simulink model "ofdm_txrx_supermimo_coded"  (Simulink 7.7)
settings: Solver=VariableStepDiscrete  SolverMode=SingleTasking  StartTime=0.0  StopTime=2*simOnly_simLength  RelTol=1e-3
PostLoadFcn:
  | ofdm_rx_supermimo_init
InitFcn:
  | ofdm_rx_supermimo_init
StartFcn:
  | ofdm_rx_supermimo_init
values from neighbouring files (symbols this model uses):
  ActionID_AFTransmit = 31    [ofdm_tx_supermimo_init.m]
  ActionID_Disabled = 0    [ofdm_tx_supermimo_init.m]
  ActionID_SetFlagA = 62    [ofdm_tx_supermimo_init.m]
  ActionID_SetFlagB = 61    [ofdm_tx_supermimo_init.m]
  BER_RAM_init_size = 2048    [ofdm_tx_supermimo_init.m]
  channel_h = [1 0; 1 0] * 0.3    [ofdm_tx_supermimo_init.m]
  CPLength = 16    [ofdm_tx_supermimo_init.m]
  longCorr_coef_i = [-4*real(LTS) 0]    [ofdm_rx_supermimo_init.m]
  longCorr_coef_q = [4*imag(LTS) 0]    [ofdm_rx_supermimo_init.m]
  max_CP_length = 16    [ofdm_tx_supermimo_init.m]
  max_num_baseRateSymbols = 31    [ofdm_tx_supermimo_init.m]
  max_num_subcarriers = 64    [ofdm_tx_supermimo_init.m]
  max_num_trainingSymbols = 15    [ofdm_tx_supermimo_init.m]
  max_numBytes = 16384    [ofdm_tx_supermimo_init.m]
  max_OFDM_symbols = 1023    [ofdm_tx_supermimo_init.m]
  modConstellation_qam16 = .75*[1 3 -1 -3]./3    [ofdm_tx_supermimo_init.m]
  modConstellation_qam64 = 0.875*[3 1 5 7 -3 -1 -5 -7]./7    [ofdm_tx_supermimo_init.m]
  modConstellation_qpsk = [1 -1]./sqrt(2)    [ofdm_tx_supermimo_init.m]
  numBaseRateSymbols = numBaseRateSymbols *2    [ofdm_tx_supermimo_init.m]
  numSubcarriers = 64    [ofdm_tx_supermimo_init.m]
  numTrainingSymbols = 2    [ofdm_tx_supermimo_init.m]
  pktTiming_count_payloadStart = pktTiming_count_lsStart + pktTiming_ls_num*pktTiming_ls_length    [ofdm_rx_supermimo_init.m]
  preamble = preamble_scale*[shortsyms_10 longsyms_2]    [ofdm_tx_supermimo_init.m]
  preamble_ROM = preamble_I_ROM + preamble_Q_ROM*2^16    [ofdm_tx_supermimo_init.m]
  RAM_init_size = max_numBytes    (= 16384)    [ofdm_tx_supermimo_init.m]
  RxDataScrambling_Seq = [40 198 78 63 82 173 102 245 48 111 172 115 147 230 216 93 72 65 62 2 205 242 122 90 128 83 105 97 73 10 5 252]    [ofdm_tx_supermimo_init.m]
  shortSymbol_time = shortSymbol_time(1:16).'    [ofdm_tx_supermimo_init.m]
  simOnly_numSamples = length(preamble)+( (numSubcarriers+CPLength)*(numTrainingSymbols + numBaseRateSymbols + numFullRateSymbols/2) )    [ofdm_tx_supermimo_init.m]
  train = [train train]    [ofdm_tx_supermimo_init.m]
  TxDataScrambling_Seq = [40 198 78 63 82 173 102 245 48 111 172 115 147 230 216 93 72 65 62 2 205 242 122 90 128 83 105 97 73 10 5 252]    [ofdm_tx_supermimo_init.m]
undefined symbols (not defined in the file or in its folder): AntA, AntB, BaseRate, Down, gcbh, numBuffs, numBytes, Up, xlBlockLoadCallback, xlGetNormalizedPeriod, xlipgetpartsetting
libraries: xbsIndex_r4 x5430
(part 11 of 49)

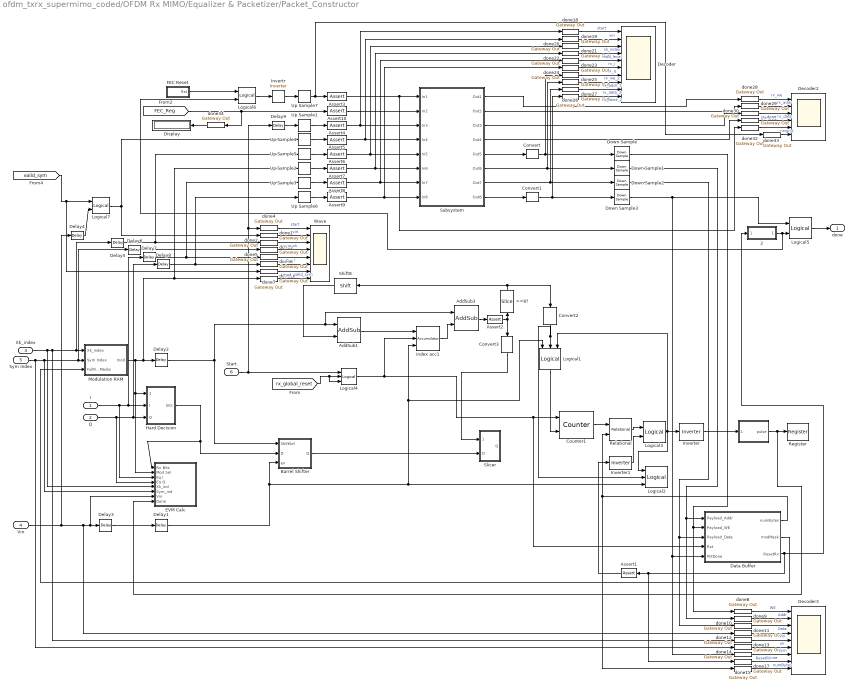
== system ofdm_txrx_supermimo_coded/OFDM Rx MIMO/Equalizer & Packetizer/Packet_Constructor ==
  I : Inport
  Q : Inport  Port=2
  Xk_index : Inport  Port=3
  Vin : Inport  Port=4
  Sym Index : Inport  Port=5
  Start : Inport  Port=6
  "  " : Subsystem  mask{period=1}
  "  2" : Subsystem  mask{period=1}
  >=8? : xbsIndex_r4/Slice  nbits=1  boolean_output=off  mode=Lower Bit Location + Width  bit1=0  base1=MSB of Input  bit0=3  base0=LSB of Input  dbl_ovrd=off  block_type=slice
      settings: infoedit="Extracts a given range of bits from each input sample and presents it at the output.  The output type is ordinarily unsigned with binary point at zero, but can be Boolean when the slice is one bit wide.<P><P>Hardware notes: In hardware this block costs nothing."  block_version=VER_STRING_GOES_HERE
  AddSub1 : xbsIndex_r4/AddSub  mode=Subtraction  use_carryin=off  use_carryout=off  en=off  latency=0  precision=User Defined  arith_type=Unsigned  n_bits=4  bin_pt=0  quantization=Truncate  overflow=Wrap  dbl_ovrd=off  use_behavioral_HDL=on  hw_selection=Fabric  pipelined=off  use_rpm=on  block_type=addsub
      settings: xl_area=[2 0 0 4 0 0 0]  block_version=VER_STRING_GOES_HERE
  AddSub3 : xbsIndex_r4/AddSub  mode=Addition  use_carryin=off  use_carryout=off  en=off  latency=0  precision=User Defined  arith_type=Unsigned  n_bits=4  bin_pt=0  quantization=Truncate  overflow=Wrap  dbl_ovrd=off  use_behavioral_HDL=on  hw_selection=Fabric  pipelined=off  use_rpm=on  block_type=addsub
      settings: xl_area=[2 0 0 4 0 0 0]  block_version=VER_STRING_GOES_HERE
  Assert1 : xbsIndex_r4/Assert  assert_type=off  type_source=Explicitly  gui_display_data_type=Fixed-point  arith_type="Signed  (2's comp)"  n_bits=16  bin_pt=14  preci_type=Single  exp_width=8  frac_width=24  assert_rate=on  rate_source=Explicitly  period=2  output_port=on  block_type=assert
      settings: infoedit=Asserts a user-defined sample rate and/or type on a signal.<P><P>Hardware notes: In hardware this block costs nothing.  block_version=VER_STRING_GOES_HERE
  Assert{3..10} (8 alike) : xbsIndex_r4/Assert  assert_type=off  type_source=Explicitly  gui_display_data_type=Fixed-point  arith_type="Signed  (2's comp)"  n_bits=16  bin_pt=14  preci_type=Single  exp_width=8  frac_width=24  assert_rate=on  rate_source=Explicitly  period=1  output_port=on  block_type=assert
      settings: infoedit=Asserts a user-defined sample rate and/or type on a signal.<P><P>Hardware notes: In hardware this block costs nothing.  block_version=10.1.3
  Assert2 : xbsIndex_r4/Assert  assert_type=off  type_source=Explicitly  gui_display_data_type=Fixed-point  arith_type="Signed  (2's comp)"  n_bits=16  bin_pt=14  preci_type=Single  exp_width=8  frac_width=24  assert_rate=on  rate_source=Explicitly  period=2  output_port=on  block_type=assert
      settings: infoedit=Asserts a user-defined sample rate and/or type on a signal.<P><P>Hardware notes: In hardware this block costs nothing.  block_version=VER_STRING_GOES_HERE
  Barrel Shifter : Subsystem
  Convert : xbsIndex_r4/Convert  gui_display_data_type=Boolean  arith_type=Boolean  n_bits=16  bin_pt=14  float_type=Single  exp_bits=8  fraction_bits=24  quantization=Truncate  overflow=Wrap  en=off  latency=0  dbl_ovrd=off  pipeline=off  block_type=convert
  Convert1 : xbsIndex_r4/Convert  gui_display_data_type=Boolean  arith_type=Boolean  n_bits=16  bin_pt=14  float_type=Single  exp_bits=8  fraction_bits=24  quantization=Truncate  overflow=Wrap  en=off  latency=0  dbl_ovrd=off  pipeline=off  block_type=convert
  Convert2 : xbsIndex_r4/Convert  gui_display_data_type=Fixed-point  arith_type=Boolean  n_bits=8  bin_pt=6  float_type=Single  exp_bits=8  fraction_bits=24  quantization=Truncate  overflow=Wrap  en=off  latency=0  dbl_ovrd=off  pipeline=off  block_type=convert
      settings: xl_area=[0 0 0 0 0 0 0]  block_version=VER_STRING_GOES_HERE
  Convert3 : xbsIndex_r4/Convert  gui_display_data_type=Fixed-point  arith_type=Unsigned  n_bits=3  bin_pt=0  float_type=Single  exp_bits=8  fraction_bits=24  quantization=Truncate  overflow=Wrap  en=off  latency=0  dbl_ovrd=off  pipeline=off  block_type=convert
      settings: xl_area=[0 0 0 0 0 0 0]  block_version=VER_STRING_GOES_HERE
  Counter1 : xbsIndex_r4/Counter  cnt_type=Free Running  cnt_to=Inf  operation=Up  start_count=0  cnt_by_val=1  arith_type=Unsigned  n_bits=ceil(log2(RAM_init_size))  bin_pt=0  load_pin=off  rst=on  en=on  explicit_period=off  period=2  dbl_ovrd=off  use_behavioral_HDL=off  implementation=Fabric  use_rpm=on  block_type=counter
      settings: xl_area=[5 10 0 10 0 0 0]  block_version=VER_STRING_GOES_HERE
  Data Buffer : Subsystem
  Decoder : Scope  NumInputPorts=10
      settings: TimeRange=14000  YMin=-1~-1~0~-1~-0.75~-1~0~0~0~-1  YMax=2~2~75~5~0.75~1~2~150~300~2  SaveName=ScopeData33
  Decoder2 : Scope  NumInputPorts=6
      settings: TimeRange=15000  YMin=-1~-1~-1~-1~-1~-1  YMax=2~2~1~2~2~2  SaveName=ScopeData35
  Decoder3 : Scope  NumInputPorts=9
      settings: TimeRange=6724.590163934425  YMin=-1~-1~-1~-1~0~0~0~-5~-5  YMax=2~2~1~2~1~3500~1~5~5  SaveName=ScopeData24
  Delay{1..9} (9 alike) : xbsIndex_r4/Delay  rst=off  infoeditControl=Selection of Reset will increase slice count due to use of real FFs and instead of SRLs  en=off  latency=1  dbl_ovrd=off  reg_retiming=off  block_type=delay
      settings: infoedit=Hardware notes: A delay line is a chain, each link of which is an SRL16 followed by a flip-flop. If register retiming is enabled, the delay line is a chain of flip-flops.  xl_area=[1 1 0 0 0 0 0]  block_version=VER_STRING_GOES_HERE
  Display : Display
  Down Sample{,1..3} (4 alike) : xbsIndex_r4/Down Sample  sample_ratio=2  sample_phase="Last Value of Frame  (most efficient)"  en=off  latency=1  dbl_ovrd=off  block_type=dsamp
      settings: block_version=10.1.3
  EVM Calc : Subsystem
  FEC Reset : Subsystem
  Hard Decision : Subsystem
  Inverter : xbsIndex_r4/Inverter  en=off  latency=0  dbl_ovrd=off  block_type=inv
      settings: xl_area=[0 0 0 0 0 0 0]  block_version=VER_STRING_GOES_HERE
  Inverter1 : xbsIndex_r4/Inverter  en=off  latency=0  dbl_ovrd=off  block_type=inv
      settings: xl_area=[0 0 0 0 0 0 0]  block_version=VER_STRING_GOES_HERE
  Invertr : xbsIndex_r4/Inverter  en=off  latency=0  dbl_ovrd=off  block_type=inv
  Logical1 : xbsIndex_r4/Logical  logical_function=AND  inputs=3  en=off  latency=0  precision=Full  arith_type=Unsigned  n_bits=8  bin_pt=2  align_bp=on  dbl_ovrd=off  block_type=logical
      settings: xl_area=[1 0 0 1 0 0 0]  block_version=VER_STRING_GOES_HERE
  Logical2 : xbsIndex_r4/Logical  logical_function=AND  inputs=3  en=off  latency=0  precision=Full  arith_type=Unsigned  n_bits=8  bin_pt=2  align_bp=on  dbl_ovrd=off  block_type=logical
      settings: xl_area=[1 0 0 1 0 0 0]  block_version=VER_STRING_GOES_HERE
  Logical3 : xbsIndex_r4/Logical  logical_function=AND  inputs=2  en=off  latency=0  precision=Full  arith_type=Unsigned  n_bits=8  bin_pt=2  align_bp=on  dbl_ovrd=off  block_type=logical
      settings: xl_area=[1 0 0 1 0 0 0]  block_version=VER_STRING_GOES_HERE
  Logical4 : xbsIndex_r4/Logical  logical_function=OR  inputs=3  en=off  latency=0  precision=Full  arith_type=Unsigned  n_bits=8  bin_pt=2  align_bp=on  dbl_ovrd=off  block_type=logical
      settings: xl_area=[4 0 0 4 0 0 0]  block_version=VER_STRING_GOES_HERE
  Logical5 : xbsIndex_r4/Logical  logical_function=OR  inputs=2  en=off  latency=0  precision=Full  arith_type=Unsigned  n_bits=8  bin_pt=2  align_bp=on  dbl_ovrd=off  block_type=logical
      settings: xl_area=[1 0 0 1 0 0 0]  block_version=VER_STRING_GOES_HERE
  Logical6 : xbsIndex_r4/Logical  logical_function=OR  inputs=2  en=off  latency=0  precision=Full  arith_type=Unsigned  n_bits=8  bin_pt=2  align_bp=on  dbl_ovrd=off  block_type=logical
      settings: xl_area=[1 0 0 1 0 0 0]  block_version=VER_STRING_GOES_HERE
  Logical7 : xbsIndex_r4/Logical  logical_function=AND  inputs=2  en=off  latency=0  precision=Full  arith_type=Unsigned  n_bits=16  bin_pt=0  align_bp=on  dbl_ovrd=off  block_type=logical
      settings: block_version=VER_STRING_GOES_HERE
  Modulation RAM : Subsystem
  Register : xbsIndex_r4/Register  init=0  rst=off  en=off  dbl_ovrd=off  block_type=register
      settings: block_version=8.2.02
  Relational : xbsIndex_r4/Relational  mode=a<b  en=off  latency=0  dbl_ovrd=off  block_type=relational
      settings: xl_area=[6 0 0 11 0 0 0]  block_version=VER_STRING_GOES_HERE
  Shift8 : xbsIndex_r4/Shift  shift_dir=Left  shift_bits=3  en=off  latency=0  precision=User Defined  arith_type=Unsigned  n_bits=4  bin_pt=0  quantization=Truncate  overflow=Wrap  dbl_ovrd=off  block_type=shift
      settings: xl_area=[0 0 0 0 0 0 0]  block_version=VER_STRING_GOES_HERE
  Slicer : Subsystem
  Subsystem : Subsystem
  Up Sample1 : xbsIndex_r4/Up Sample  sample_ratio=4  copy_samples=off  en=off  latency=0  dbl_ovrd=off  block_type=usamp
      settings: infoedit="Up samples input data.  Inserted values  can be zeros or copies of the most recent input sample.<P><P>Hardware notes: No hardware is needed if inserted values are copies of the input sample; otherwise, a mux and single bit flip-flop are used."  block_version=10.1.3
  Up Sample{2..3,6..7} (4 alike) : xbsIndex_r4/Up Sample  sample_ratio=2  copy_samples=on  en=off  latency=0  dbl_ovrd=off  block_type=usamp
      settings: infoedit="Up samples input data.  Inserted values  can be zeros or copies of the most recent input sample.<P><P>Hardware notes: No hardware is needed if inserted values are copies of the input sample; otherwise, a mux and single bit flip-flop are used."  block_version=10.1.3
  Up Sample4 : xbsIndex_r4/Up Sample  sample_ratio=2  copy_samples=off  en=off  latency=0  dbl_ovrd=off  block_type=usamp
      settings: infoedit="Up samples input data.  Inserted values  can be zeros or copies of the most recent input sample.<P><P>Hardware notes: No hardware is needed if inserted values are copies of the input sample; otherwise, a mux and single bit flip-flop are used."  block_version=10.1.3
  Up Sample5 : xbsIndex_r4/Up Sample  sample_ratio=2  copy_samples=off  en=off  latency=0  dbl_ovrd=off  block_type=usamp
      settings: infoedit="Up samples input data.  Inserted values  can be zeros or copies of the most recent input sample.<P><P>Hardware notes: No hardware is needed if inserted values are copies of the input sample; otherwise, a mux and single bit flip-flop are used."  block_version=10.1.3
  Wave : Scope  NumInputPorts=8
      settings: TimeRange=18000  YMin=-1~-1~-1~24.7~0.00168152~-0.75~0~0  YMax=2~2~1~27.3~0.00185852~0.75~2~5  SaveName=ScopeData21
  done{1..34} (34 alike) : xbsIndex_r4/Gateway Out  inherit_from_input=off  hdl_port=off  timing_constraint=None  locs_specified=off  LOCs={}  UseAsDAC=off  DACChannel='1'  block_type=gatewayout
      settings: infoedit="Gateway out block.  Converts Xilinx fixed point inputs into ouputs of type Simulink integer, double, or fixed point.<P><P>Hardware notes:  In hardware these blocks become top level output ports or are discarded, depending on how they are configured."  xl_area=[0 0 0 0 1 0 0]  block_version=VER_STRING_GOES_HERE
  index acc1 : xbsIndex_r4/Accumulator  operation=Add  n_bits=4  overflow=Wrap  scale=1  rst=on  hasbypass=off  en=on  dbl_ovrd=off  use_behavioral_HDL=on  implementation=Fabric  block_type=accum
      settings: infoedit="Adder or subtractor-based accumulator.   Output type and binary point position match the input.<P><P>Hardware notes: When \"Reinitialize with input 'b' on reset\" is selected, the accumulator is forced to run at the system rate even if the input 'b' is running at a slower rate."  xl_area=[2 4 0 4 0 0 0]  block_version=VER_STRING_GOES_HERE
  done : Outport
  wires:
  Delay2/1 -> AddSub1/1, AddSub3/1, Barrel Shifter/1(ShiftSel)
  Data Buffer/1(numBytes) -> Relational/2, done17/1
  Logical4/1 -> index acc1/2, Counter1/1, Data Buffer/4(Rst)
  AddSub1/1 -> index acc1/1
  Shift8/1 -> AddSub1/2
  index acc1/1 -> AddSub3/2
  AddSub3/1 -> Assert2/1
  >=8?/1 -> Shift8/1, Convert2/1
  Barrel Shifter/1(Q) -> Slicer/2(D)
  "  "/1(pulse) -> Register/1, EVM Calc/8(Done)
  Inverter/1 -> "  "/1(signal)
  Convert2/1 -> Logical1/2, Logical2/2
  Logical1/1 -> Counter1/2
  Logical3/1 -> Logical2/1, Logical1/3, Inverter/1
  Counter1/1 -> Relational/1
  Convert3/1 -> Slicer/1(SliceSel)
  Modulation RAM/1(mod) -> Hard Decision/1(Mod Sel), EVM Calc/2(Mod Sel), Delay2/1, done16/1, Up Sample2/1
  Xk_index/1 -> EVM Calc/5(Xk_ind), Modulation RAM/1(Xk_index), Delay5/1, done12/1
  Sym Index/1 -> EVM Calc/6(Sym_ind), Delay6/1, Modulation RAM/2(Sym Index), done13/1
  Hard Decision/1(bits) -> Barrel Shifter/2(D), EVM Calc/1(Rx Bits)
  I/1 -> EVM Calc/3(Eq I), Hard Decision/2(I), Delay7/1
  Q/1 -> EVM Calc/4(Eq Q), Hard Decision/3(Q), Delay8/1
  Start/1 -> Logical4/1, done4/1, Delay9/1
  [rx_global_reset] -> Logical4/2, Logical4/3
  Assert2/1 -> Convert3/1, >=8?/1
  Vin/1 -> Delay3/1, EVM Calc/7(Vin), done11/1, Delay4/1
  Delay1/1 -> Barrel Shifter/3(en), index acc1/3, Logical1/1, Logical2/3
  Delay3/1 -> Delay1/1
  Data Buffer/2(modMask) -> Modulation RAM/3(FullRateMasks)
  Relational/1 -> Logical3/1
  Inverter1/1 -> Logical3/2
  Assert1/1 -> Inverter1/1
  Data Buffer/3(ResetRx) -> Assert1/1, done15/1, "  2"/1(signal)
  done4/1 -> Wave/1  "start"
  done1/1 -> Wave/2  "vin"
  done2/1 -> Wave/3  "xk"
  done3/1 -> Wave/4  "sym"
  done5/1 -> Wave/5  "I"
  done6/1 -> Wave/6  "Q"
  done7/1 -> Wave/7  "valid_sym"
  done8/1 -> Decoder3/1  "WE"
  done9/1 -> Decoder3/2  "Addr"
  done10/1 -> Decoder3/3  "Data"
  done11/1 -> Decoder3/4  "Vin"
  done12/1 -> Decoder3/5  "xk"
  done13/1 -> Decoder3/6  "Sym"
  done14/1 -> Decoder3/7  "done"
  done15/1 -> Decoder3/8  "ResetRx"
  done16/1 -> Wave/8  "mod"
  done17/1 -> Decoder3/9  "numBytes"
  Delay5/1 -> done2/1, Up Sample5/1
  Delay6/1 -> done3/1
  Delay7/1 -> done5/1, Up Sample3/1
  Delay8/1 -> done6/1, Up Sample6/1
  Invertr/1 -> Up Sample7/1
  Up Sample1/1 -> Assert4/1
  Assert4/1 -> done18/1, Subsystem/3(In3)
  Delay9/1 -> Up Sample1/1
  Up Sample4/1 -> Assert5/1
  Assert5/1 -> done19/1, Subsystem/4(In4)
  Assert6/1 -> done20/1, Subsystem/5(In5)
  Up Sample5/1 -> Assert6/1
  Up Sample2/1 -> Assert7/1
  Assert7/1 -> done21/1, Subsystem/6(In6)
  Up Sample3/1 -> Assert8/1
  Assert8/1 -> done22/1, Subsystem/7(In7)
  Up Sample6/1 -> Assert9/1
  Assert9/1 -> done23/1, Subsystem/8(In8)
  [FEC_Reg] -> Assert10/1, done34/1
  Assert10/1 -> Subsystem/2(In2)
  Subsystem/5(Out5) -> Convert/1
  Subsystem/6(Out6) -> done25/1, Down Sample1/1
  Subsystem/7(Out7) -> done26/1, Down Sample2/1
  done18/1 -> Decoder/1  "start"
  done20/1 -> Decoder/3  "xk_index"
  done21/1 -> Decoder/4  "mod_level"
  done22/1 -> Decoder/5  "rx_i"
  done23/1 -> Decoder/6  "rx_q"
  done24/1 -> Decoder/7  "rx_we_2"
  done25/1 -> Decoder/8  "rx_addr_2"
  done26/1 -> Decoder/9  "rx_data_2"
  done27/1 -> Decoder/10  "rx_done_2"
  done19/1 -> Decoder/2  "vin"
  done28/1 -> Decoder2/1  "rx_we"
  Subsystem/1(Out1) -> done28/1
  Subsystem/2(Out2) -> done29/1
  done29/1 -> Decoder2/2  "rx_addr"
  done30/1 -> Decoder2/3  "rx_data"
  Subsystem/3(Out3) -> done30/1
  done31/1 -> Decoder2/4  "rx_done"
  Subsystem/4(Out4) -> done31/1
  done32/1 -> Decoder2/5  "nrst"
  Down Sample/1 -> Data Buffer/2(Payload_WE), done8/1
  Down Sample1/1 -> Data Buffer/1(Payload_Addr), done9/1
  Down Sample2/1 -> Data Buffer/3(Payload_Data), done10/1
  Down Sample3/1 -> Data Buffer/5(PktDone), done14/1, Logical5/1
  Assert3/1 -> done32/1, Subsystem/1(In1)
  done33/1 -> Decoder2/6  "grst"
  Up Sample7/1 -> Assert3/1, done33/1
  FEC Reset/1(Rst) -> Logical6/1
  "  2"/1(pulse) -> Logical6/2, Logical5/2
  Logical5/1 -> done/1
  Logical6/1 -> Invertr/1
  [valid_sym] -> Logical7/1, done7/1
  Delay4/1 -> Logical7/2
  Logical7/1 -> Up Sample4/1, done1/1
  done34/1 -> Display/1
  Convert1/1 -> done27/1, Down Sample3/1
  Convert/1 -> Down Sample/1, done24/1
  Subsystem/8(Out8) -> Convert1/1

== system ofdm_txrx_supermimo_coded/OFDM Rx MIMO/Equalizer & Packetizer/Scaling ==
  note: This block scales the equalized constellation so it can be easily sliced/demapped for hard decisions,
  Eq-I : Inport
  Eq-Q : Inport  Port=2
  Xk_index : Inport  Port=3
  Vin : Inport  Port=4
  Sym Index : Inport  Port=5
  " To_UFix16_11" : xbsIndex_r4/Reinterpret  force_arith_type=on  arith_type=Unsigned  force_bin_pt=on  bin_pt=11  block_type=cast
      settings: infoedit="Changes type of samples without altering their binary representation.<P><P>Hardware notes: In hardware this block costs nothing.<P><P>Example:  Suppose the input is 6 bits wide, signed, with 2 fractional bits, and the output is forced to unsigned with 0 fractional bits.  Then an input of -2.0 (1110.00 in binary 2's complement) becomes an output of 56 (111000 in binary)."  block_version=VER_STRING_GOES_HERE
  16LSB : xbsIndex_r4/Slice  nbits=16  boolean_output=off  mode=Lower Bit Location + Width  bit1=0  base1=LSB of Input  bit0=0  base0=LSB of Input  dbl_ovrd=off  block_type=slice
      settings: infoedit="Extracts a given range of bits from each input sample and presents it at the output.  The output type is ordinarily unsigned with binary point at zero, but can be Boolean when the slice is one bit wide.<P><P>Hardware notes: In hardware this block costs nothing."  block_version=VER_STRING_GOES_HERE
  16MSB : xbsIndex_r4/Slice  nbits=16  boolean_output=off  mode=Lower Bit Location + Width  bit1=0  base1=LSB of Input  bit0=16  base0=LSB of Input  dbl_ovrd=off  block_type=slice
      settings: infoedit="Extracts a given range of bits from each input sample and presents it at the output.  The output type is ordinarily unsigned with binary point at zero, but can be Boolean when the slice is one bit wide.<P><P>Hardware notes: In hardware this block costs nothing."  block_version=VER_STRING_GOES_HERE
  1LSB : xbsIndex_r4/Slice  nbits=1  boolean_output=off  mode=Lower Bit Location + Width  bit1=0  base1=MSB of Input  bit0=0  base0=LSB of Input  dbl_ovrd=off  block_type=slice
      settings: infoedit="Extracts a given range of bits from each input sample and presents it at the output.  The output type is ordinarily unsigned with binary point at zero, but can be Boolean when the slice is one bit wide.<P><P>Hardware notes: In hardware this block costs nothing."  block_version=VER_STRING_GOES_HERE
  Delay1 : xbsIndex_r4/Delay  rst=off  infoeditControl=Selection of Reset will increase slice count due to use of real FFs and instead of SRLs  en=off  latency=1  dbl_ovrd=off  reg_retiming=off  block_type=delay
      settings: infoedit=Hardware notes: A delay line is a chain, each link of which is an SRL16 followed by a flip-flop. If register retiming is enabled, the delay line is a chain of flip-flops.  xl_area=[0, 0, 0, 0, 0, 0, 0]  block_version=VER_STRING_GOES_HERE
  Delay3 : xbsIndex_r4/Delay  rst=off  infoeditControl=Selection of Reset will increase slice count due to use of real FFs and instead of SRLs  en=off  latency=1  dbl_ovrd=off  reg_retiming=off  block_type=delay
      settings: infoedit=Hardware notes: A delay line is a chain, each link of which is an SRL16 followed by a flip-flop. If register retiming is enabled, the delay line is a chain of flip-flops.  xl_area=[0, 0, 0, 0, 0, 0, 0]  block_version=VER_STRING_GOES_HERE
  Delay4 : xbsIndex_r4/Delay  rst=off  infoeditControl=Selection of Reset will increase slice count due to use of real FFs and instead of SRLs  en=off  latency=1  dbl_ovrd=off  reg_retiming=off  block_type=delay
      settings: infoedit=Hardware notes: A delay line is a chain, each link of which is an SRL16 followed by a flip-flop. If register retiming is enabled, the delay line is a chain of flip-flops.  xl_area=[0, 0, 0, 0, 0, 0, 0]  block_version=VER_STRING_GOES_HERE
  Down Sample1 : xbsIndex_r4/Down Sample  sample_ratio=2  sample_phase="Last Value of Frame  (most efficient)"  en=off  latency=1  dbl_ovrd=off  block_type=dsamp
      settings: block_version=VER_STRING_GOES_HERE
  Mult : xbsIndex_r4/Mult  precision=User Defined  arith_type="Signed  (2's comp)"  n_bits=symbol_unmap_nb  bin_pt=symbol_unmap_bp  quantization="Round  (unbiased: +/- Inf)"  overflow=Saturate  en=off  latency=1  dbl_ovrd=off  use_behavioral_HDL=off  opt=Speed  use_embedded=on  optimum_pipeline=off  pipeline=on  use_rpm=off  placement_style=Rectangular shape  block_type=mult
      settings: infoedit=Hardware notes: To use the internal pipeline stage of the dedicated multiplier you must select 'Pipeline for maximum performance'.  xl_area=[12 0 0 20 0 1 0]  block_version=VER_STRING_GOES_HERE
  Mult1 : xbsIndex_r4/Mult  precision=User Defined  arith_type="Signed  (2's comp)"  n_bits=symbol_unmap_nb  bin_pt=symbol_unmap_bp  quantization="Round  (unbiased: +/- Inf)"  overflow=Saturate  en=off  latency=1  dbl_ovrd=off  use_behavioral_HDL=off  opt=Speed  use_embedded=on  optimum_pipeline=off  pipeline=on  use_rpm=off  placement_style=Rectangular shape  block_type=mult
      settings: infoedit=Hardware notes: To use the internal pipeline stage of the dedicated multiplier you must select 'Pipeline for maximum performance'.  xl_area=[12 0 0 20 0 1 0]  block_version=VER_STRING_GOES_HERE
  Mux : xbsIndex_r4/Mux  inputs=2  en=off  latency=0  precision=Full  arith_type=Unsigned  n_bits=16  bin_pt=14  quantization=Truncate  overflow=Wrap  dbl_ovrd=off  block_type=mux
      settings: block_version=VER_STRING_GOES_HERE
  To_UFix16_11 : xbsIndex_r4/Reinterpret  force_arith_type=on  arith_type=Unsigned  force_bin_pt=on  bin_pt=11  block_type=cast
      settings: infoedit="Changes type of samples without altering their binary representation.<P><P>Hardware notes: In hardware this block costs nothing.<P><P>Example:  Suppose the input is 6 bits wide, signed, with 2 fractional bits, and the output is forced to unsigned with 0 fractional bits.  Then an input of -2.0 (1110.00 in binary 2's complement) becomes an output of 56 (111000 in binary)."  block_version=VER_STRING_GOES_HERE
  I : Outport
  Q : Outport  Port=2
  " Xk_index" : Outport  Port=3
  Vout : Outport  Port=4
  " Sym Index" : Outport  Port=5
  tag scope: EQ_I=global, EQ_Q=global
  wires:
  Eq-Q/1 -> Mult1/2
  Eq-I/1 -> Mult/2
  Mult1/1 -> [EQ_Q], Q/1
  Mult/1 -> [EQ_I], I/1
  Mux/1 -> Mult1/1, Mult/1
  1LSB/1 -> Mux/1
  " To_UFix16_11"/1 -> Mux/3
  To_UFix16_11/1 -> Mux/2
  Xk_index/1 -> Delay1/1
  Delay1/1 -> " Xk_index"/1
  Vin/1 -> Delay3/1
  Delay3/1 -> Vout/1
  Sym Index/1 -> Delay4/1, 1LSB/1
  [RxReg_PostEQScaling] -> Down Sample1/1
  16LSB/1 -> To_UFix16_11/1
  16MSB/1 -> " To_UFix16_11"/1
  Down Sample1/1 -> 16LSB/1, 16MSB/1
  Delay4/1 -> " Sym Index"/1

== system ofdm_txrx_supermimo_coded/OFDM Rx MIMO/Equalizer & Packetizer/posedge ==
  note: Rising Edge Detection
  D : Inport
  Delay : xbsIndex_r4/Delay  rst=off  infoeditControl=Selection of Reset will increase slice count due to use of real FFs and instead of SRLs  en=off  latency=1  dbl_ovrd=off  reg_retiming=off  block_type=delay
      settings: infoedit=Hardware notes: A delay line is a chain, each link of which is an SRL16 followed by a flip-flop. If register retiming is enabled, the delay line is a chain of flip-flops.  xl_area=[1 1 0 0 0 0 0]  block_version=VER_STRING_GOES_HERE
  Inverter : xbsIndex_r4/Inverter  en=off  latency=0  dbl_ovrd=off  block_type=inv
      settings: xl_area=[0 0 0 0 0 0 0]  block_version=VER_STRING_GOES_HERE
  Logical1 : xbsIndex_r4/Logical  logical_function=AND  inputs=2  en=off  latency=0  precision=Full  arith_type=Unsigned  n_bits=8  bin_pt=2  align_bp=on  dbl_ovrd=off  block_type=logical
      settings: xl_area=[1 0 0 1 0 0 0]  block_version=VER_STRING_GOES_HERE
  Q : Outport
  wires:
  Delay/1 -> Inverter/1
  Inverter/1 -> Logical1/1
  Logical1/1 -> Q/1
  D/1 -> Delay/1, Logical1/2

== system ofdm_txrx_supermimo_coded/OFDM Rx MIMO/FFT & Chan Est/Addr Gen1 ==
  FFT Done : Inport
  Payload : Inport  Port=2
  NotTraining : Inport  Port=3
  1MSB : xbsIndex_r4/Slice  nbits=1  boolean_output=on  mode=Upper Bit Location + Width  bit1=0  base1=MSB of Input  bit0=0  base0=MSB of Input  dbl_ovrd=off  block_type=slice
      settings: infoedit="Extracts a given range of bits from each input sample and presents it at the output.  The output type is ordinarily unsigned with binary point at zero, but can be Boolean when the slice is one bit wide.<P><P>Hardware notes: In hardware this block costs nothing."  block_version=VER_STRING_GOES_HERE
  6LSB : xbsIndex_r4/Slice  nbits=6  boolean_output=off  mode=Lower Bit Location + Width  bit1=0  base1=LSB of Input  bit0=0  base0=LSB of Input  dbl_ovrd=off  block_type=slice
      settings: infoedit="Extracts a given range of bits from each input sample and presents it at the output.  The output type is ordinarily unsigned with binary point at zero, but can be Boolean when the slice is one bit wide.<P><P>Hardware notes: In hardware this block costs nothing."  block_version=VER_STRING_GOES_HERE
  Constant1 : xbsIndex_r4/Constant  const=numSubcarriers*2-1  gui_display_data_type=Fixed-point  arith_type=Unsigned  n_bits=ceil(log2(numSubcarriers*2))  bin_pt=0  preci_type=Single  exp_width=8  frac_width=24  explicit_period=off  period=1  equ=P=C  opselect=C  inp2=PCIN>>17  opr=+  inp1=P  carry=CIN  dbl_ovrd=off  block_type=constant
      settings: block_version=VER_STRING_GOES_HERE
  Copy_Downsample : Subsystem
  Counter : xbsIndex_r4/Counter  cnt_type=Free Running  cnt_to=127  operation=Up  start_count=0  cnt_by_val=1  arith_type=Unsigned  n_bits=ceil(log2(numSubcarriers*2))  bin_pt=0  load_pin=off  rst=on  en=on  explicit_period=off  period=2  dbl_ovrd=off  use_behavioral_HDL=off  implementation=Fabric  use_rpm=on  block_type=counter
      settings: xl_area=[6 12 0 12 0 0 0]  block_version=VER_STRING_GOES_HERE
  Delay : xbsIndex_r4/Delay  rst=off  infoeditControl=Selection of Reset will increase slice count due to use of real FFs and instead of SRLs  en=off  latency=1  dbl_ovrd=off  reg_retiming=off  block_type=delay
      settings: block_version=10.1.2
  Delay1 : xbsIndex_r4/Delay  rst=off  infoeditControl=Selection of Reset will increase slice count due to use of real FFs and instead of SRLs  en=off  latency=1  dbl_ovrd=off  reg_retiming=off  block_type=delay
      settings: block_version=10.1.2
  Inverter2 : xbsIndex_r4/Inverter  en=off  latency=0  dbl_ovrd=off  block_type=inv
      settings: block_version=VER_STRING_GOES_HERE
  Inverter3 : xbsIndex_r4/Inverter  en=off  latency=0  dbl_ovrd=off  block_type=inv
      settings: block_version=VER_STRING_GOES_HERE
  Logical1 : xbsIndex_r4/Logical  logical_function=AND  inputs=3  en=off  latency=0  precision=Full  arith_type=Unsigned  n_bits=8  bin_pt=2  align_bp=on  dbl_ovrd=off  block_type=logical
      settings: xl_area=[0, 0, 0, 0, 0, 0, 0]  block_version=VER_STRING_GOES_HERE
  Logical10 : xbsIndex_r4/Logical  logical_function=AND  inputs=2  en=off  latency=0  precision=Full  arith_type=Unsigned  n_bits=8  bin_pt=2  align_bp=on  dbl_ovrd=off  block_type=logical
      settings: xl_area=[0, 0, 0, 0, 0, 0, 0]  block_version=VER_STRING_GOES_HERE
  Logical11 : xbsIndex_r4/Logical  logical_function=AND  inputs=2  en=off  latency=0  precision=Full  arith_type=Unsigned  n_bits=8  bin_pt=2  align_bp=on  dbl_ovrd=off  block_type=logical
      settings: xl_area=[0, 0, 0, 0, 0, 0, 0]  block_version=VER_STRING_GOES_HERE
  Logical2 : xbsIndex_r4/Logical  logical_function=OR  inputs=2  en=off  latency=0  precision=Full  arith_type=Unsigned  n_bits=8  bin_pt=2  align_bp=on  dbl_ovrd=off  block_type=logical
      settings: xl_area=[4 0 0 4 0 0 0]  block_version=VER_STRING_GOES_HERE
  Logical3 : xbsIndex_r4/Logical  logical_function=OR  inputs=2  en=off  latency=0  precision=Full  arith_type=Unsigned  n_bits=8  bin_pt=2  align_bp=on  dbl_ovrd=off  block_type=logical
      settings: xl_area=[0, 0, 0, 0, 0, 0, 0]  block_version=VER_STRING_GOES_HERE
  Logical4 : xbsIndex_r4/Logical  logical_function=OR  inputs=2  en=off  latency=0  precision=Full  arith_type=Unsigned  n_bits=8  bin_pt=2  align_bp=on  dbl_ovrd=off  block_type=logical
      settings: xl_area=[0, 0, 0, 0, 0, 0, 0]  block_version=VER_STRING_GOES_HERE
  Logical6 : xbsIndex_r4/Logical  logical_function=AND  inputs=3  en=off  latency=0  precision=Full  arith_type=Unsigned  n_bits=8  bin_pt=2  align_bp=on  dbl_ovrd=off  block_type=logical
      settings: xl_area=[0, 0, 0, 0, 0, 0, 0]  block_version=VER_STRING_GOES_HERE
  Logical7 : xbsIndex_r4/Logical  logical_function=NAND  inputs=2  en=off  latency=0  precision=Full  arith_type=Unsigned  n_bits=8  bin_pt=2  align_bp=on  dbl_ovrd=off  block_type=logical
      settings: xl_area=[1 0 0 1 0 0 0]  block_version=VER_STRING_GOES_HERE
  Oscillator : Subsystem
  Output Sym Counter : Subsystem
  Rd Delay : Subsystem
  Relational : xbsIndex_r4/Relational  mode=a<b  en=off  latency=0  dbl_ovrd=off  block_type=relational
      settings: block_version=VER_STRING_GOES_HERE
  S-R Latch1 : Subsystem
  SymIndex_out : Outport
  Rd Addr : Outport  Port=2
  Vout : Outport  Port=3
  wires:
  Counter/1 -> 6LSB/1, Relational/1, 1MSB/1
  6LSB/1 -> Rd Addr/1, Output Sym Counter/4(Read Addr)
  Constant1/1 -> Relational/2
  [regRx_SISOmode] -> Logical7/1
  1MSB/1 -> Logical7/2
  Payload/1 -> Output Sym Counter/3(Payload), Inverter2/1, Logical6/3
  Inverter2/1 -> Oscillator/2(rst), Logical2/1
  NotTraining/1 -> Logical1/3, Output Sym Counter/2(Not Training)
  Oscillator/1(Q) -> Logical1/2
  S-R Latch1/1(D) -> Logical10/1
  Logical1/1 -> Delay/1
  Logical10/1 -> Counter/2, Delay1/1, Logical4/1
  Relational/1 -> Logical10/2, Inverter3/1
  Inverter3/1 -> Logical11/2
  [rx_global_reset] -> Logical2/2
  Logical2/1 -> S-R Latch1/2(R), Logical3/1
  Copy_Downsample/1(Q) -> Output Sym Counter/1(FFT Done), Rd Delay/1(D)
  Delay/1 -> S-R Latch1/1(S), Logical11/1
  Logical6/1 -> Vout/1
  Logical7/1 -> Logical6/1
  Output Sym Counter/1(Sym Index) -> SymIndex_out/1
  Logical11/1 -> Logical3/2
  Logical3/1 -> Counter/1
  Delay1/1 -> Logical4/2
  Logical4/1 -> Logical6/2
  FFT Done/1 -> Copy_Downsample/1(D)
  Rd Delay/1(Q) -> Oscillator/1(en), Logical1/1

== system ofdm_txrx_supermimo_coded/OFDM Rx MIMO/FFT & Chan Est/Addr_Generator ==
  note: The FFT core begins requesting input samples immediately
      | after its Start input is asserted. If Start is asserted again before
      | the first transform is complete, a second transform begins
      | immediately after the first. This is how we sequence the pairs
      | of transforms for the incoming OFDM symbols multiplexed between
      | antennas. The FFT is started as soon as the incoming sample stream
      | passes sample number (numSubcarriers/2)+symbolTimingOffset.
      | This is the lowest latency scheme, loading the FFT core with the
      | input OFDM symbol exactly 1 clock cycle after it's available.
  note: The memory read address is the FFT input index plus an offset in [0,max_cyclic_prefix]. This is how we strip
      | the cyclic prefix from the incoming sample stream- the FFT only gets numSubcarrier samples.
  Payload : Inport
  FFT Xn_index : Inport  Port=2
  AddSub : xbsIndex_r4/AddSub  mode=Addition  use_carryin=off  use_carryout=off  en=off  latency=0  precision=User Defined  arith_type=Unsigned  n_bits=ceil(log2(max_num_subcarriers))+1  bin_pt=0  quantization=Truncate  overflow=Wrap  dbl_ovrd=off  use_behavioral_HDL=on  hw_selection=Fabric  pipelined=off  use_rpm=on  block_type=addsub
      settings: xl_area=[4 0 0 6 0 0 0]  block_version=VER_STRING_GOES_HERE
  Assert : xbsIndex_r4/Assert  assert_type=on  type_source=Explicitly  gui_display_data_type=Fixed-point  arith_type=Boolean  n_bits=16  bin_pt=14  preci_type=Single  exp_width=8  frac_width=24  assert_rate=off  rate_source=Explicitly  period=1  output_port=on  block_type=assert
      settings: infoedit=Asserts a user-defined sample rate and/or type on a signal.<P><P>Hardware notes: In hardware this block costs nothing.  block_version=VER_STRING_GOES_HERE
  Convert : xbsIndex_r4/Convert  gui_display_data_type=Fixed-point  arith_type=Unsigned  n_bits=ceil(log2(max_num_subcarriers))+1  bin_pt=0  float_type=Single  exp_bits=8  fraction_bits=24  quantization=Truncate  overflow=Wrap  en=off  latency=0  dbl_ovrd=off  pipeline=off  block_type=convert
      settings: block_version=VER_STRING_GOES_HERE
  Delay : xbsIndex_r4/Delay  rst=off  infoeditControl=Selection of Reset will increase slice count due to use of real FFs and instead of SRLs  en=off  latency=64  dbl_ovrd=off  reg_retiming=off  block_type=delay
      settings: infoedit=Hardware notes: A delay line is a chain, each link of which is an SRL16 followed by a flip-flop. If register retiming is enabled, the delay line is a chain of flip-flops.  block_version=VER_STRING_GOES_HERE
  Down Sample : xbsIndex_r4/Down Sample  sample_ratio=2  sample_phase="Last Value of Frame  (most efficient)"  en=off  latency=1  dbl_ovrd=off  block_type=dsamp
      settings: block_version=VER_STRING_GOES_HERE
  Initial Read Addr Timing Calc : Subsystem
  Inverter : xbsIndex_r4/Inverter  en=off  latency=0  dbl_ovrd=off  block_type=inv
      settings: block_version=VER_STRING_GOES_HERE
  Inverter1 : xbsIndex_r4/Inverter  en=off  latency=0  dbl_ovrd=off  block_type=inv
      settings: block_version=VER_STRING_GOES_HERE
  Logical1 : xbsIndex_r4/Logical  logical_function=OR  inputs=3  en=off  latency=0  precision=Full  arith_type=Unsigned  n_bits=16  bin_pt=0  align_bp=on  dbl_ovrd=off  block_type=logical
      settings: block_version=VER_STRING_GOES_HERE
  Logical2 : xbsIndex_r4/Logical  logical_function=OR  inputs=2  en=off  latency=0  precision=Full  arith_type=Unsigned  n_bits=16  bin_pt=0  align_bp=on  dbl_ovrd=off  block_type=logical
      settings: block_version=VER_STRING_GOES_HERE
  Logical3 : xbsIndex_r4/Logical  logical_function=OR  inputs=2  en=off  latency=0  precision=Full  arith_type=Unsigned  n_bits=16  bin_pt=0  align_bp=on  dbl_ovrd=off  block_type=logical
      settings: block_version=VER_STRING_GOES_HERE
  Logical4 : xbsIndex_r4/Logical  logical_function=AND  inputs=3  en=off  latency=0  precision=Full  arith_type=Unsigned  n_bits=16  bin_pt=0  align_bp=on  dbl_ovrd=off  block_type=logical
      settings: block_version=VER_STRING_GOES_HERE
  Logical5 : xbsIndex_r4/Logical  logical_function=OR  inputs=2  en=off  latency=0  precision=Full  arith_type=Unsigned  n_bits=16  bin_pt=0  align_bp=on  dbl_ovrd=off  block_type=logical
      settings: block_version=VER_STRING_GOES_HERE
  MSB : xbsIndex_r4/Slice  nbits=1  boolean_output=on  mode=Upper Bit Location + Width  bit1=0  base1=MSB of Input  bit0=0  base0=LSB of Input  dbl_ovrd=off  block_type=slice
      settings: infoedit="Extracts a given range of bits from each input sample and presents it at the output.  The output type is ordinarily unsigned with binary point at zero, but can be Boolean when the slice is one bit wide.<P><P>Hardware notes: In hardware this block costs nothing."  block_version=VER_STRING_GOES_HERE
  Oscillator : Subsystem
  Posedge : Subsystem
  Register : xbsIndex_r4/Register  init=0  rst=on  en=on  dbl_ovrd=off  block_type=register
      settings: block_version=VER_STRING_GOES_HERE
  Relational : xbsIndex_r4/Relational  mode=a=b  en=off  latency=0  dbl_ovrd=off  block_type=relational
      settings: block_version=VER_STRING_GOES_HERE
  Relational1 : xbsIndex_r4/Relational  mode=a=b  en=off  latency=0  dbl_ovrd=off  block_type=relational
      settings: block_version=VER_STRING_GOES_HERE
  Samp Counter : xbsIndex_r4/Counter  cnt_type=Free Running  cnt_to=1  operation=Up  start_count=0  cnt_by_val=1  arith_type=Unsigned  n_bits=ceil(log2(max_num_subcarriers + max_CP_length))  bin_pt=0  load_pin=off  rst=on  en=on  explicit_period=off  period=1  dbl_ovrd=off  use_behavioral_HDL=off  implementation=Fabric  use_rpm=on  block_type=counter
      settings: xl_area=[5 7 0 9 0 0 0]  block_version=VER_STRING_GOES_HERE
  Up Sample1 : xbsIndex_r4/Up Sample  sample_ratio=4  copy_samples=on  en=off  latency=0  dbl_ovrd=off  block_type=usamp
      settings: infoedit="Up samples input data.  Inserted values  can be zeros or copies of the most recent input sample.<P><P>Hardware notes: No hardware is needed if inserted values are copies of the input sample; otherwise, a mux and single bit flip-flop are used."  block_version=VER_STRING_GOES_HERE
  Up Sample2 : xbsIndex_r4/Up Sample  sample_ratio=4  copy_samples=on  en=off  latency=0  dbl_ovrd=off  block_type=usamp
      settings: infoedit="Up samples input data.  Inserted values  can be zeros or copies of the most recent input sample.<P><P>Hardware notes: No hardware is needed if inserted values are copies of the input sample; otherwise, a mux and single bit flip-flop are used."  block_version=VER_STRING_GOES_HERE
  Up Sample6 : xbsIndex_r4/Up Sample  sample_ratio=2  copy_samples=on  en=off  latency=0  dbl_ovrd=off  block_type=usamp
      settings: infoedit="Up samples input data.  Inserted values  can be zeros or copies of the most recent input sample.<P><P>Hardware notes: No hardware is needed if inserted values are copies of the input sample; otherwise, a mux and single bit flip-flop are used."  block_version=VER_STRING_GOES_HERE
  negedge : Subsystem
  negedge1 : Subsystem
  Write Addr : Outport
  Read Addr : Outport  Port=2
  Ant Sel : Outport  Port=3
  Wr Slot : Outport  Port=4
  FFT Start : Outport  Port=5
  wires:
  negedge1/1(Q) -> Logical1/1
  Up Sample2/1 -> Logical4/2
  Logical4/1 -> FFT Start/1
  [localReset] -> Register/2
  Assert/1 -> Inverter/1
  Inverter/1 -> Register/1
  Register/1 -> Assert/1, Ant Sel/1
  negedge/1(Q) -> Register/3
  MSB/1 -> negedge/1(D)
  Logical2/1 -> Logical4/3
  Up Sample1/1 -> Relational1/1
  FFT Xn_index/1 -> Convert/1, MSB/1
  Down Sample/1 -> Relational/1
  Relational1/1 -> Logical2/1, Delay/1
  AddSub/1 -> Read Addr/1
  [rx_global_reset_DV2] -> Logical1/3
  [localReset] -> Logical3/2, Oscillator/2(rst)
  Logical3/1 -> Samp Counter/1
  Payload/1 -> negedge1/1(D), Samp Counter/2, Up Sample2/1, Posedge/1(D)
  Logical1/1 -> [localReset]
  Posedge/1(Q) -> Logical1/2
  Relational/1 -> Logical3/1, Oscillator/1(en)
  Samp Counter/1 -> Up Sample1/1, Write Addr/1, Relational/2
  [regRX_sampsPerSymMinusOne] -> Down Sample/1
  [regRx_symbolTimingOffset] -> Up Sample6/1
  Convert/1 -> AddSub/1
  Up Sample6/1 -> AddSub/2
  Initial Read Addr Timing Calc/1(D) -> Relational1/2
  Delay/1 -> Logical2/2
  Oscillator/1(Q) -> Wr Slot/1, Logical5/2
  Logical5/1 -> Logical4/1
  [regRx_AlamoutiMode] -> Inverter1/1
  Inverter1/1 -> Logical5/1

== system ofdm_txrx_supermimo_coded/OFDM Rx MIMO/FFT & Chan Est/Channel Estimation & Pilot Processing ==
  note: Naming conventions:
      | Antennas are labaled A and B
      | H_A-A is channel between TxA and RxA
      | H_A-B is channel between TxA and RxB
      | H_B-A is channel between TxB and RxA
      | H_B-B is channel between TxB and RxB
  I : Inport
  Q : Inport  Port=2
  Xk Index : Inport  Port=3
  Vin : Inport  Port=4
  Sym Index : Inport  Port=5
  Est Index : Inport  Port=6
  Rst : Inport  Port=7
  Actual Trainig Window : Subsystem
  Bus Creator : Subsystem
  Chan Est Buffer & Mag Calc : Subsystem
  Delay1 : xbsIndex_r4/Delay  rst=off  infoeditControl=Selection of Reset will increase slice count due to use of real FFs and instead of SRLs  en=off  latency=1  dbl_ovrd=off  reg_retiming=on  block_type=delay
      settings: infoedit=Hardware notes: A delay line is a chain, each link of which is an SRL16 followed by a flip-flop. If register retiming is enabled, the delay line is a chain of flip-flops.  xl_area=[0, 0, 0, 0, 0, 0, 0]  block_version=VER_STRING_GOES_HERE
  Delay{2,4..11} (9 alike) : xbsIndex_r4/Delay  rst=off  infoeditControl=Selection of Reset will increase slice count due to use of real FFs and instead of SRLs  en=off  latency=1  dbl_ovrd=off  reg_retiming=off  block_type=delay
      settings: infoedit=Hardware notes: A delay line is a chain, each link of which is an SRL16 followed by a flip-flop. If register retiming is enabled, the delay line is a chain of flip-flops.  xl_area=[0, 0, 0, 0, 0, 0, 0]  block_version=VER_STRING_GOES_HERE
  Delay3 : xbsIndex_r4/Delay  rst=off  infoeditControl=Selection of Reset will increase slice count due to use of real FFs and instead of SRLs  en=off  latency=1  dbl_ovrd=off  reg_retiming=on  block_type=delay
      settings: infoedit=Hardware notes: A delay line is a chain, each link of which is an SRL16 followed by a flip-flop. If register retiming is enabled, the delay line is a chain of flip-flops.  xl_area=[0, 0, 0, 0, 0, 0, 0]  block_version=VER_STRING_GOES_HERE
  Down Sample : xbsIndex_r4/Down Sample  sample_ratio=2  sample_phase="Last Value of Frame  (most efficient)"  en=off  latency=1  dbl_ovrd=off  block_type=dsamp
      settings: block_version=10.1.2
  Downsamplng : Subsystem
  H_A-A Estimate Buffer : Subsystem
  H_A-B Estimate Buffer : Subsystem
  H_B-A Estimate Buffer : Subsystem
  H_B-B Estimate Buffer : Subsystem
  Inverter : xbsIndex_r4/Inverter  en=off  latency=1  dbl_ovrd=off  block_type=inv
      settings: block_version=VER_STRING_GOES_HERE
  Logical1 : xbsIndex_r4/Logical  logical_function=OR  inputs=2  en=off  latency=0  precision=Full  arith_type=Unsigned  n_bits=8  bin_pt=2  align_bp=on  dbl_ovrd=off  block_type=logical
      settings: xl_area=[1 0 0 1 0 0 0]  block_version=VER_STRING_GOES_HERE
  Logical2 : xbsIndex_r4/Logical  logical_function=OR  inputs=2  en=off  latency=0  precision=Full  arith_type=Unsigned  n_bits=8  bin_pt=2  align_bp=on  dbl_ovrd=off  block_type=logical
      settings: xl_area=[1 0 0 1 0 0 0]  block_version=VER_STRING_GOES_HERE
  Mult by training : Subsystem
  Mux3 : xbsIndex_r4/Mux  inputs=2  en=off  latency=0  precision=Full  arith_type="Signed  (2's comp)"  n_bits=8  bin_pt=2  quantization=Truncate  overflow=Wrap  dbl_ovrd=off  block_type=mux
      settings: xl_area=[0, 0, 0, 0, 0, 0, 0]  block_version=VER_STRING_GOES_HERE
  Pilot Processing : Subsystem
  Training Symbol : xbsIndex_r4/ROM  depth=length(train)/2  initVector=sign(train)  distributed_mem=Block RAM  rst=off  init_reg=0  en=off  latency=1  gui_display_data_type=Fixed-point  arith_type="Signed  (2's comp)"  n_bits=2  bin_pt=1  preci_type=Single  exp_width=8  frac_width=24  dbl_ovrd=off  optimize=Area  use_rpm=off  block_type=sprom
      settings: xl_area=[0, 0, 0, 0, 0, 0, 0]  block_version=VER_STRING_GOES_HERE
  Training? : Subsystem
  WE Gen : Subsystem
  done{1..14,23} (15 alike) : xbsIndex_r4/Gateway Out  inherit_from_input=off  hdl_port=off  timing_constraint=None  locs_specified=off  LOCs={}  UseAsDAC=off  DACChannel='1'  block_type=gatewayout
      settings: infoedit="Gateway out block.  Converts Xilinx fixed point inputs into ouputs of type Simulink integer, double, or fixed point.<P><P>Hardware notes:  In hardware these blocks become top level output ports or are discarded, depending on how they are configured."  xl_area=[0 0 0 0 1 0 0]  block_version=VER_STRING_GOES_HERE
  H-I : Outport
  H-Q : Outport  Port=2
  Phase I : Outport  Port=3
  Phase Q : Outport  Port=4
  Est Vout : Outport  Port=5
  wires:
  Delay5/1 -> H_A-B Estimate Buffer/4(Est_Reset), H_B-B Estimate Buffer/4(Est_Reset), Logical1/2, Logical2/2
  Delay2/1 -> Mux3/3, H_A-A Estimate Buffer/5(FFT_Addr), Pilot Processing/4(Xk_Index), H_B-A Estimate Buffer/5(FFT_Addr)
  Est Index/1 -> Mux3/2, Delay7/1, Chan Est Buffer & Mag Calc/10(SubCar Index)
  Delay10/1 -> Delay11/1
  done23/1 -> ?
  done9/1 -> ?
  done2/1 -> ?
  done8/1 -> ?
  done7/1 -> ?
  done6/1 -> ?
  done3/1 -> ?
  done5/1 -> ?
  done4/1 -> ?
  done1/1 -> ?
  H_B-B Estimate Buffer/2(EstQ) -> Downsamplng/8(BB-Q)
  H_B-B Estimate Buffer/1(EstI) -> Downsamplng/7(BB-I)
  H_B-A Estimate Buffer/4(EstQ) -> Downsamplng/6(BA-Q)
  H_B-A Estimate Buffer/3(EstI) -> Downsamplng/5(BA-I)
  H_A-B Estimate Buffer/2(EstQ) -> Downsamplng/4(AB-Q)
  H_A-B Estimate Buffer/1(EstI) -> Downsamplng/3(AB-I)
  H_A-A Estimate Buffer/4(EstQ) -> Downsamplng/2(AA-Q)
  H_A-A Estimate Buffer/3(EstI) -> Downsamplng/1(AA-I)
  Delay11/1 -> Down Sample/1
  Inverter/1 -> Delay10/1
  Delay7/1 -> done2/1
  Delay9/1 -> WE Gen/1(Vin)
  Vin/1 -> Delay9/1, Actual Trainig Window/1(H Vin), done10/1
  Delay8/1 -> Mult by training/2(AI)
  Delay6/1 -> Mult by training/1(AR)
  Delay3/1 -> Mux3/1, WE Gen/3(Train), Actual Trainig Window/2(Train Sym), done11/1
  Delay1/1 -> WE Gen/2(Sym Index), Pilot Processing/5(Sym_Index)
  Mult by training/2(PI) -> H_A-B Estimate Buffer/2(Q), H_A-A Estimate Buffer/2(Q), H_B-B Estimate Buffer/2(Q), H_B-A Estimate Buffer/2(Q)
  Training?/2(Training) -> Delay3/1, Inverter/1
  WE Gen/2(Seq1) -> H_A-B Estimate Buffer/3(Est_WE)
  WE Gen/4(Seq3) -> H_B-B Estimate Buffer/3(Est_WE)
  WE Gen/3(Seq2) -> H_B-A Estimate Buffer/3(Est_WE)
  WE Gen/1(Seq0) -> H_A-A Estimate Buffer/3(Est_WE)
  Mux3/1 -> H_B-B Estimate Buffer/5(Xk_Index), H_A-B Estimate Buffer/5(Xk_Index), H_A-A Estimate Buffer/6(EQ_Addr), H_B-A Estimate Buffer/6(EQ_Addr)
  Mult by training/1(PR) -> H_A-B Estimate Buffer/1(I), H_A-A Estimate Buffer/1(I), H_B-A Estimate Buffer/1(I), H_B-B Estimate Buffer/1(I)
  Xk Index/1 -> Training Symbol/1, Delay4/1, done14/1
  Sym Index/1 -> Training?/1(SynIndex), Delay1/1, done13/1
  Q/1 -> Delay8/1, Pilot Processing/3(Q)
  I/1 -> Delay6/1, Pilot Processing/2(I)
  Delay4/1 -> Delay2/1
  Training Symbol/1 -> Mult by training/3(BR)
  Down Sample/1 -> Est Vout/1, Chan Est Buffer & Mag Calc/9(Est Vin), done23/1
  Bus Creator/1(I) -> H-I/1
  Bus Creator/2(Q) -> H-Q/1
  Downsamplng/1(" AA-I") -> Bus Creator/1(AA-I), Chan Est Buffer & Mag Calc/1(AA-I), done9/1
  Downsamplng/2(" AA-Q") -> Bus Creator/2(AA-Q), Chan Est Buffer & Mag Calc/2(AA-Q), done4/1
  Downsamplng/3(" AB-I") -> Bus Creator/3(AB-I), Chan Est Buffer & Mag Calc/3(AB-I), done5/1
  Downsamplng/4(" AB-Q") -> Bus Creator/4(AB-Q), Chan Est Buffer & Mag Calc/4(AB-Q), done3/1
  Downsamplng/5(" BA-I") -> Bus Creator/5(BA-I), Chan Est Buffer & Mag Calc/5(BA-I), done6/1
  Downsamplng/6(" BA-Q") -> Bus Creator/6(BA-Q), Chan Est Buffer & Mag Calc/6(BA-Q), done1/1
  Downsamplng/7(" BB-I") -> Bus Creator/7(BB-I), Chan Est Buffer & Mag Calc/7(BB-I), done7/1
  Downsamplng/8(" BB-Q") -> Bus Creator/8(BB-Q), Chan Est Buffer & Mag Calc/8(BB-Q), done8/1
  Training?/1(FirstSym) -> Delay5/1
  Pilot Processing/1(Phase I) -> Phase I/1
  H_A-A Estimate Buffer/1(EstI_fast) -> Pilot Processing/7(HAA_I)
  H_A-A Estimate Buffer/2(EstQ_fast) -> Pilot Processing/8(HAA_Q)
  H_B-A Estimate Buffer/2(EstQ_fast) -> Pilot Processing/10(HBA_Q)
  H_B-A Estimate Buffer/1(EstI_fast) -> Pilot Processing/9(HBA_I)
  Actual Trainig Window/1(Train) -> Pilot Processing/6(Train), done12/1
  Pilot Processing/2(Phase Q) -> Phase Q/1
  Logical2/1 -> H_A-A Estimate Buffer/4(Est_Reset)
  [ChanEstMagCheck_MaskAA] -> Logical2/1
  Logical1/1 -> H_B-A Estimate Buffer/4(Est_Reset)
  [ChanEstMagCheck_MaskBA] -> Logical1/1
  done10/1 -> ?
  done11/1 -> ?
  done12/1 -> ?
  done13/1 -> ?
  done14/1 -> ?
  Rst/1 -> Pilot Processing/1(Rst)
